simulink model "nonlinear_double_track_model"  (R2024b)
settings: Solver=VariableStepAuto  StartTime=0.0  StopTime=5  FixedStep=0.01  RelTol=1e-4
undefined symbols (not defined in the file or in its folder): a, b, balance_bar_ratio_front, brake_pedal_motion_ratio, cg_height, disc_radius_front, disc_radius_rear, front_mass_distribution, g, gear_ratio, Jeq, Ki_yaw, Kp_yaw, mass_total, max_pedal_force, mc_diameter_front, mc_diameter_rear, num_pistons_front, num_pistons_rear, pad_friction_front, pad_friction_rear, pad_height_front, pad_height_rear, piston_radius_front, piston_radius_rear, static_toe_front, static_toe_rear, tire_loaded_radius, tire_mu, tire_mu_correction_factor, tlltd, toe_deflection_rear, track_width_front, track_width_rear, wheelbase, yaw_polar_inertia
libraries: simulink_extras x12, checkpacklib x1, slpidlib x1
(part 1 of 6)
settings Model: Creator=bglen  LastModifiedBy=37rst
settings RTWCC: GPUComputeCapability=3.5

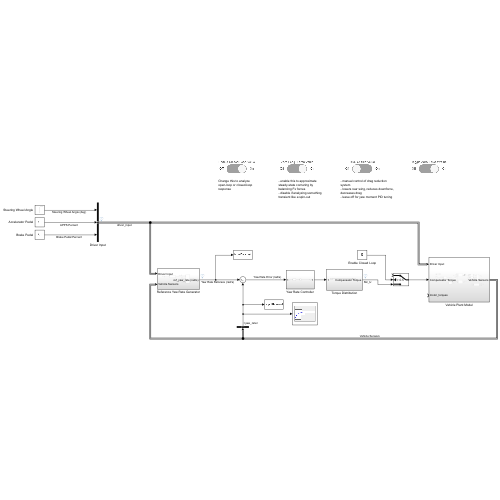
[thumbnail: Simulink's stored preview of the top-level diagram]
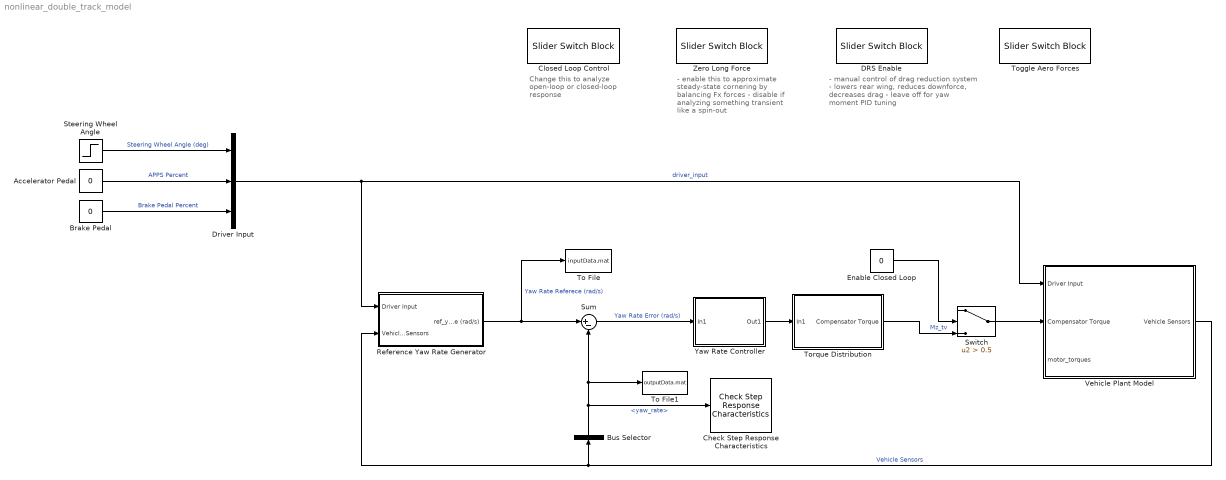
== system nonlinear_double_track_model ==
  note: - enable this to approximate
      | steady-state cornering by
      | balancing Fx forces
      | - disable if analyzing something
      | transient like a spin-out
  note: Change this to analyze
      | open-loop or closed-loop
      | response
  note: - manual control of drag reduction
      | system
      | - lowers rear wing, reduces downforce,
      | decreases drag
      | - leave off for yaw moment PID tuning
  Accelerator Pedal : Constant  Value=0
  Brake Pedal : Constant  Value=0
  Bus Selector : BusSelector  OutputSignals=yaw_rate
  Check Step Response Characteristics : checkpacklib/Check Step Response  Characteristics  InstantiateOnLoad=on  enabled=on  stopWhenAssertionFail=off  export=off  ViewDlgPos=[]  OpenViewOnLoad=off  LaunchViewOnOpen=off  TimeUnits=seconds  EnableStepResponseBound=on  InitialValue=0  FinalValue=.426  StepTime=1  RiseTime=1  PercentRise=80  SettlingTime=2  PercentSettling=1  PercentOvershoot=10  PercentUndershoot=1
      settings: LibrarySourceBlock=|
      | sdolib/Signal Constraints/Check Step Response
      | Characteristics
  Closed Loop Control : SliderSwitchBlock
      settings: Values={{'Off', 'On'}, [1 0]}
  DRS Enable : SliderSwitchBlock
      settings: Values={{'Off', 'On'}, [0 1]}
  Driver Input : BusCreator  Inputs=3
  Enable Closed Loop : Constant  Value=0
  Reference Yaw Rate Generator : Subsystem
  Steering Wheel Angle : Step  After=90  SampleTime=0
  Sum : Sum  Inputs=|+-
  Switch : Switch  Criteria=u2 > Threshold  Threshold=0.5  SaturateOnIntegerOverflow=off  InputSameDT=off
  To File : ToFile  Filename=inputData.mat  MatrixName=inputData
  To File1 : ToFile  Filename=outputData.mat  MatrixName=outputData
  Toggle Aero Forces : SliderSwitchBlock
      settings: Values={{'Off', 'On'}, [0 -1]}
  Torque Distribution : Subsystem
  Vehicle Plant Model : Subsystem
  Yaw Rate Controller : Subsystem
  Zero Long Force : SliderSwitchBlock
      settings: Values={{'Off', 'On'}, [0 1]}
  wires:
  Sum/1 -> Yaw Rate Controller/1(In1)  "Yaw Rate Error (rad/s)"
  Accelerator Pedal/1 -> Driver Input/2  "APPS Percent"
  Steering Wheel Angle/1 -> Driver Input/1  "Steering Wheel Angle (deg)"
  Brake Pedal/1 -> Driver Input/3  "Brake Pedal Percent"
  Driver Input/1 -> Reference Yaw Rate Generator/1(Driver Input), Vehicle Plant Model/1(Driver Input)  "driver_input"
  Vehicle Plant Model/1(Vehicle Sensors) -> Reference Yaw Rate Generator/2(Vehicle Sensors), Bus Selector/1  "Vehicle Sensors"
  Bus Selector/1 -> Check Step Response Characteristics/1, Sum/2, To File1/1  "<yaw_rate>"
  Yaw Rate Controller/1(Out1) -> Torque Distribution/1(In1)
  Reference Yaw Rate Generator/1(ref_yaw_rate (rad/s)) -> To File/1, Sum/1  "Yaw Rate Referece (rad/s)"
  Switch/1 -> Vehicle Plant Model/2(Compensator Torque)
  Torque Distribution/1(Compensator Torque) -> Switch/3  "Mz_tv"
  Enable Closed Loop/1 -> Switch/2

== system nonlinear_double_track_model/Reference Yaw Rate Generator ==
  Driver Input : Inport
  Vehicle Sensors : Inport  Port=2
  Bus Selector : BusSelector  OutputSignals=body_velocity_x
  Bus Selector1 : BusSelector  OutputSignals=Steering Wheel Angle (deg)
  MATLAB Function : MATLAB Function  TreatAsAtomicUnit=on
  ZR25 Steering System : Subsystem
  g : Constant  Value=g
  l : Constant  Value=wheelbase
  mu : Constant  Value=tire_mu
  sigma : Constant  Value=tire_mu_correction_factor
  ref_yaw_rate (rad/s) : Outport
  wires:
  MATLAB Function/1(ref_yaw_rate) -> ref_yaw_rate (rad/s)/1
  mu/1 -> MATLAB Function/3(mu)
  sigma/1 -> MATLAB Function/4(sigma)
  l/1 -> MATLAB Function/5(wheelbase)
  g/1 -> MATLAB Function/6(g)
  Vehicle Sensors/1 -> Bus Selector/1
  Bus Selector/1 -> MATLAB Function/1(velocity)  "<body_velocity_x>"
  Driver Input/1 -> Bus Selector1/1
  Bus Selector1/1 -> ZR25 Steering System/1(Steering input at the rack)  "<Steering Wheel Angle (deg)>"
  ZR25 Steering System/1(Steering Angles (rad)) -> MATLAB Function/2(steer_angles)  "Front Steering Angles (rad)"

== system nonlinear_double_track_model/Torque Distribution ==
  In1 : Inport
  Compensator Torque : Outport
  wires:
  In1/1 -> Compensator Torque/1

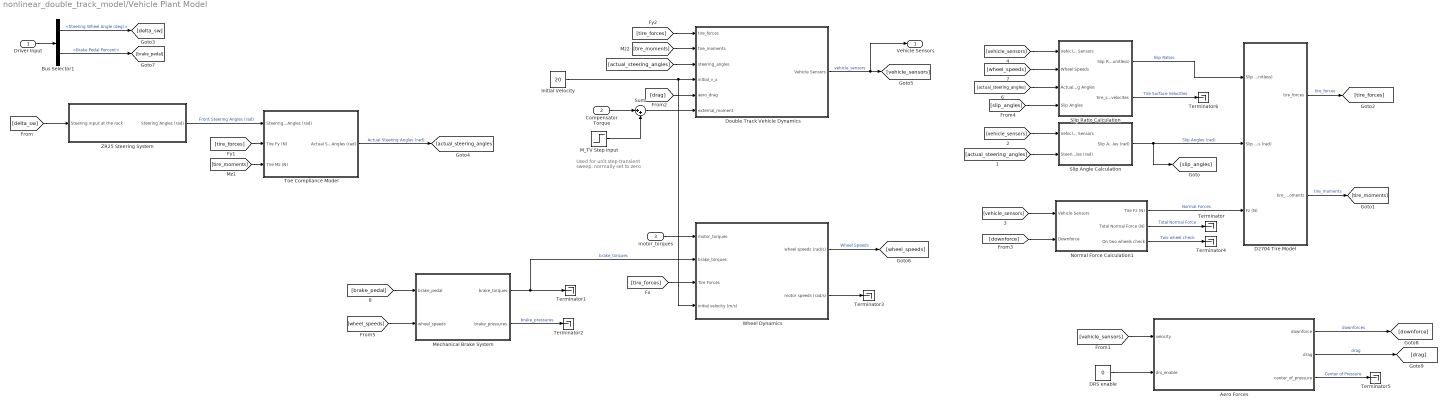
== system nonlinear_double_track_model/Vehicle Plant Model ==
  note: Used for unit step transient sweep, normally set to zero
  Driver Input : Inport
  Compensator Torque : Inport  Port=2
  motor_torques : Inport  Port=3
  Aero Forces : Subsystem
      mask Display: image('big_wing.png');
  Bus Selector1 : BusSelector  OutputSignals=Steering Wheel Angle (deg),Brake Pedal Percent
  D2704 Tire Model : Subsystem
  DRS enable : Constant  Value=0
  Double Track Vehicle Dynamics : Subsystem
      mask Display: image('double_track_diagram.png');
  Initial Velocity : Constant  Value=20
  M_TV Step Input : Step  After=0  SampleTime=0
  Mechanical Brake System : Subsystem
  Normal Force Calculation1 : Subsystem
  Slip Angle Calculation : Subsystem
  Slip Ratio Calculation : Subsystem
  Sum : Sum  Inputs=|++
  Terminator{,1..6} (7 alike) : Terminator
  Toe Compliance Model : Subsystem
  Wheel Dynamics : Subsystem
  ZR25 Steering System : Subsystem
  Vehicle Sensors : Outport
  wires:
  Slip Angle Calculation/1(Slip Angles (rad)) -> [slip_angles], D2704 Tire Model/2(Slip Angles (rad))  "Slip Angles (rad)"
  Normal Force Calculation1/2(Total Normal Force (N)) -> Terminator/1  "Total Normal Force"
  Normal Force Calculation1/1(Tire Fz (N)) -> D2704 Tire Model/3(Fz (N))  "Normal Forces"
  ZR25 Steering System/1(Steering Angles (rad)) -> Toe Compliance Model/1(Steering Angles (rad))  "Front Steering Angles (rad)"
  Initial Velocity/1 -> Wheel Dynamics/4(initial velocity (m/s)), Double Track Vehicle Dynamics/4(initial_v_x)
  Normal Force Calculation1/3(On two wheels check) -> Terminator4/1  "Two wheel check"
  D2704 Tire Model/2(tire_moments) -> [tire_moments]  "tire_moments"
  [tire_moments] -> Double Track Vehicle Dynamics/2(tire_moments)
  D2704 Tire Model/1(tire_forces) -> [tire_forces]  "tire_forces"
  [tire_forces] -> Double Track Vehicle Dynamics/1(tire_forces)
  Driver Input/1 -> Bus Selector1/1
  Bus Selector1/1 -> [delta_sw]  "<Steering Wheel Angle (deg)>"
  [delta_sw] -> ZR25 Steering System/1(Steering input at the rack)
  M_TV Step Input/1 -> Sum/2
  Compensator Torque/1 -> Sum/1
  Sum/1 -> Double Track Vehicle Dynamics/6(external_moment)
  Toe Compliance Model/1(Actual Steering Angles (rad)) -> [actual_steering_angles]  "Actual Steering Angles (rad)"
  [tire_forces] -> Toe Compliance Model/2(Tire Fy (N))
  [tire_moments] -> Toe Compliance Model/3(Tire Mz (N))
  [actual_steering_angles] -> Double Track Vehicle Dynamics/3(steering_angles)
  [actual_steering_angles] -> Slip Angle Calculation/2(Steering Angles (rad))
  [vehicle_sensors] -> Slip Angle Calculation/1(Vehicle Sensors)
  [vehicle_sensors] -> Normal Force Calculation1/1(Vehicle Sensors)
  [vehicle_sensors] -> Slip Ratio Calculation/1(Vehicle Sensors)
  Double Track Vehicle Dynamics/1(Vehicle Sensors) -> Vehicle Sensors/1, [vehicle_sensors]  "vehicle_sensors"
  [actual_steering_angles] -> Slip Ratio Calculation/3(Actual Steering Angles)
  Wheel Dynamics/1(wheel speeds (rad/s)) -> [wheel_speeds]  "Wheel Speeds"
  [wheel_speeds] -> Slip Ratio Calculation/2(Wheel Speeds)
  motor_torques/1 -> Wheel Dynamics/1(motor_torques)
  Bus Selector1/2 -> [brake_pedal]  "<Brake Pedal Percent>"
  [brake_pedal] -> Mechanical Brake System/1(brake_pedal)
  Mechanical Brake System/1(brake_torques) -> Wheel Dynamics/2(brake_torques), Terminator1/1  "brake_torques"
  Mechanical Brake System/2(brake_pressures) -> Terminator2/1  "brake_pressures"
  Wheel Dynamics/2(motor speeds (rad/s)) -> Terminator3/1
  Aero Forces/1(downforce) -> [downforce]  "downforces"
  [vehicle_sensors] -> Aero Forces/1(velocity)
  DRS enable/1 -> Aero Forces/2(drs_enable)
  Aero Forces/2(drag) -> [drag]  "drag"
  Aero Forces/3(center_of_pressure) -> Terminator5/1  "Center of Pressure"
  [drag] -> Double Track Vehicle Dynamics/5(aero_drag)
  [downforce] -> Normal Force Calculation1/2(Downforce)
  [slip_angles] -> Slip Ratio Calculation/4(Slip Angles)
  Slip Ratio Calculation/2(tire_surface_velocites) -> Terminator6/1  "Tire Surface Velocities"
  Slip Ratio Calculation/1(Slip Ratios (unitless)) -> D2704 Tire Model/1(Slip Ratios (unitless))  "Slip Ratios"
  [tire_forces] -> Wheel Dynamics/3(Tire Forces)
  [wheel_speeds] -> Mechanical Brake System/2(wheel_speeds)

== system nonlinear_double_track_model/Yaw Rate Controller ==
  In1 : Inport
  PID Controller : slpidlib/PID Controller  Controller=PI  Form=Parallel  TimeDomain=Continuous-time  UseExternalTs=off  SampleTime=-1  IntegratorMethod=Forward Euler  FilterMethod=Forward Euler  ControllerParametersSource=internal  P=Kp_yaw  I=Ki_yaw  UseKiTs=off  D=0  UseExternalDerivativeSource=off  N=100  UseFilter=on  TunerSelectOption=Transfer Function Based (PID Tuner App)  InitialConditionSource=internal  InitialConditionForIntegrator=0  InitialConditionForFilter=0  DifferentiatorICPrevScaledInput=0  ExternalReset=none  TrackingMode=off  Kt=1  LimitOutput=off  SatLimitsSource=internal  UpperSaturationLimit=7000  LowerSaturationLimit=-7000  LinearizeAsGain=on  AntiWindupMode=back-calculation  Kb=1  LimitIntegrator=off  UpperIntegratorSaturationLimit=inf  LowerIntegratorSaturationLimit=-inf  POutMin=[]  POutMax=[]  IOutMin=[]  IOutMax=[]  DOutMin=[]  DOutMax=[]  NOutMin=[]  NOutMax=[]  SumOutMin=[]  SumOutMax=[]  SaturationOutMin=[]  SaturationOutMax=[]  PParamMin=[]  PParamMax=[]  IParamMin=[]  IParamMax=[]  DParamMin=[]  DParamMax=[]  NParamMin=[]  NParamMax=[]  KbParamMin=[]  KbParamMax=[]  KtParamMin=[]  KtParamMax=[]  KbOutMin=[]  KbOutMax=[]  KtOutMin=[]  KtOutMax=[]  IntegratorOutMin=[]  IntegratorOutMax=[]  FilterOutMin=[]  FilterOutMax=[]  SumI1OutMin=[]  SumI1OutMax=[]  SumI2OutMin=[]  SumI2OutMax=[]  SumI3OutMin=[]  SumI3OutMax=[]  SumI4OutMin=[]  SumI4OutMax=[]  SumDOutMin=[]  SumDOutMax=[]  DifferentiatorOutMin=[]  DifferentiatorOutMax=[]  FilterDiffOutCoefMin=[]  FilterDiffOutCoefMax=[]  ReciprocalOutMin=[]  ReciprocalOutMax=[]  SumDenOutMin=[]  SumDenOutMax=[]  SumNumOutMin=[]  SumNumOutMax=[]  DivideOutMin=[]  DivideOutMax=[]  UdiffTsProdOutMin=[]  UdiffTsProdOutMax=[]  NTsProdOutMin=[]  NTsProdOutMax=[]  UintegralTsProdOutMin=[]  UintegralTsProdOutMax=[]  UngainTsProdOutMin=[]  UngainTsProdOutMax=[]  ClampingZeroOutDataTypeStr=Inherit: Inherit via back propagation  ClampingZeroOutMin=[]  ClampingZeroOutMax=[]  FilterDenConstantOutDataTypeStr=Inherit: Inherit via back propagation  FilterDenConstantOutMin=[]  FilterDenConstantOutMax=[]  PCopyOutDataTypeStr=Inherit: Inherit via back propagation  PCopyOutMin=[]  PCopyOutMax=[]  NCopyOutDataTypeStr=Inherit: Inherit via back propagation  NCopyOutMin=[]  NCopyOutMax=[]  IntegratorContinuousStateAttributes=''  IntegratorStateMustResolveToSignalObject=off  IntegratorRTWStateStorageClass=Auto  FilterContinuousStateAttributes=''  FilterStateMustResolveToSignalObject=off  FilterRTWStateStorageClass=Auto  ParallelPVariant=InternalParameters  IdealPVariant=Disabled  IVariant=InternalParameters  DVariant=Disabled  IntegratorVariant=Continuous  SatVariant=Disabled  AWVariant=Passthrough  PCopyVariant=Disabled  TRVariant=Disabled  FdbkBlocksVariant=Forward  IdealPFdbkVariant=Disabled  SatFdbkVariant=Disabled  DerivativeFilterVariant=Disabled  NVariant=Disabled  NCopyVariant=Disabled_SignalSpec  FilterICVariant=Disabled  IntegratorICVariant=InternalICs  ExternalResetVariant=Disabled  TRSumVariant=Passthrough  SumFdbkVariant=Disabled  SumVariant=Sum_PI  TsampFilterVariant=InternalTs  TsampNgainVariant=Passthrough  TsampIntegralVariant=Disabled_SignalSpec  ExternalSaturationOutDataTypeStr=Inherit: Same as second input  DerivativeSourceVariant=Disabled  PreIntVariant=InternalPreInt
  Saturation : Saturate  UpperLimit=7000  LowerLimit=-7000
  Out1 : Outport
  wires:
  In1/1 -> PID Controller/1
  PID Controller/1 -> Saturation/1
  Saturation/1 -> Out1/1

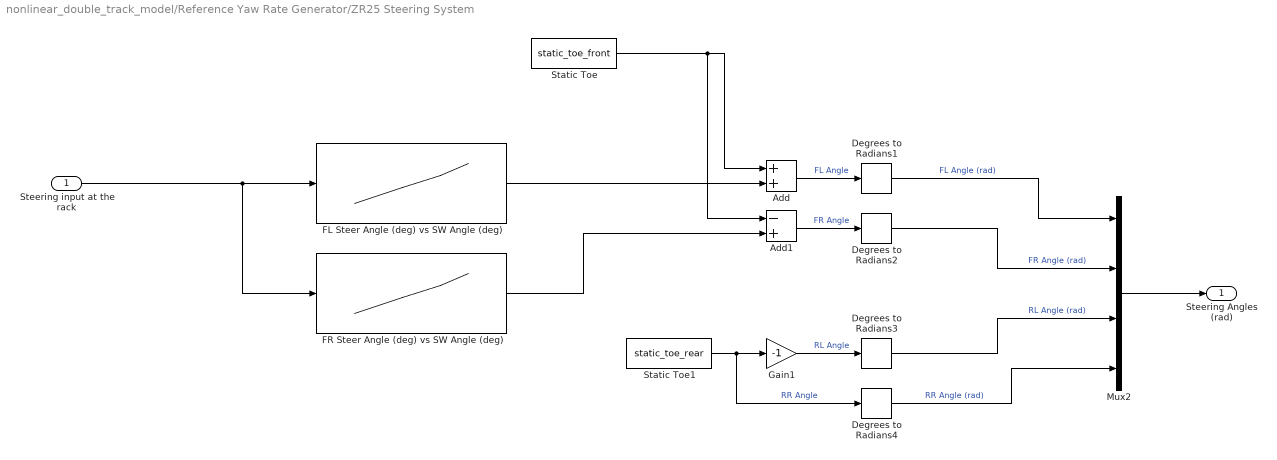
== system nonlinear_double_track_model/Reference Yaw Rate Generator/ZR25 Steering System ==
  Steering input at the rack : Inport
  Add : Sum
  Add1 : Sum  Inputs=-+
  Degrees to Radians{1..4} (4 alike) : simulink_extras/Transformations/Degrees to Radians
  FL Steer Angle (deg) vs SW Angle (deg) : Lookup_n-D  NumberOfTableDimensions=1  BreakpointsForDimension1=[-180;-162;-144;-126;-108;-90;-72;-54;-36;-18;0;18;36;54;72;90;108;126;144;162;180]  Table=[-37.683;-33.549;-29.57;-25.708;-21.934;-18.224;-14.558;-10.918;-7.289;-3.654;0;3.689;7.428;11.234;15.126;19.127;23.261;27.563;32.073;36.847;41.964]  InternalRulePriority=Speed  RndMeth=Simplest
  FR Steer Angle (deg) vs SW Angle (deg) : Lookup_n-D  NumberOfTableDimensions=1  BreakpointsForDimension1=[-180;-162;-144;-126;-108;-90;-72;-54;-36;-18;0;18;36;54;72;90;108;126;144;162;180]  Table=[-41.964;-36.847;-32.073;-27.563;-23.261;-19.126;-15.126;-11.234;-7.428;-3.689;0;3.654;7.289;10.918;14.558;18.225;21.934;25.708;29.57;33.549;37.683]  InternalRulePriority=Speed  RndMeth=Simplest
  Gain1 : Gain  Gain=-1
  Mux2 : Mux
  Static Toe : Constant  Value=static_toe_front
  Static Toe1 : Constant  Value=static_toe_rear
  Steering Angles (rad) : Outport
  wires:
  Steering input at the rack/1 -> FR Steer Angle (deg) vs SW Angle (deg)/1, FL Steer Angle (deg) vs SW Angle (deg)/1
  Add1/1 -> Degrees to Radians2/1  "FR Angle"
  Add/1 -> Degrees to Radians1/1  "FL Angle"
  Static Toe/1 -> Add/1, Add1/1
  Static Toe1/1 -> Gain1/1, Degrees to Radians4/1  "RR Angle"
  Gain1/1 -> Degrees to Radians3/1  "RL Angle"
  Degrees to Radians1/1 -> Mux2/1  "FL Angle (rad)"
  Degrees to Radians2/1 -> Mux2/2  "FR Angle (rad)"
  Degrees to Radians3/1 -> Mux2/3  "RL Angle (rad)"
  Degrees to Radians4/1 -> Mux2/4  "RR Angle (rad)"
  Mux2/1 -> Steering Angles (rad)/1
  FL Steer Angle (deg) vs SW Angle (deg)/1 -> Add/2
  FR Steer Angle (deg) vs SW Angle (deg)/1 -> Add1/2

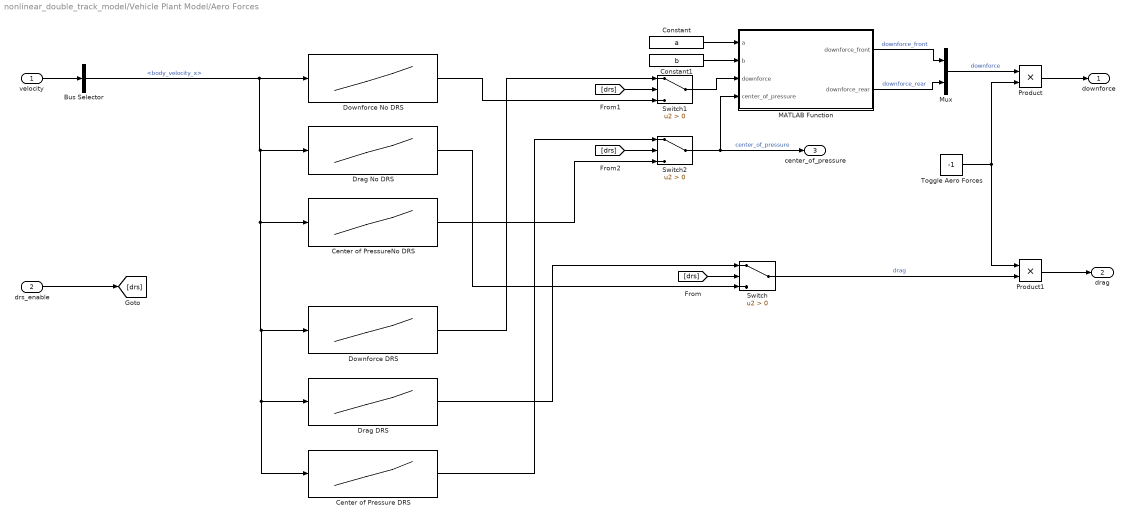
== system nonlinear_double_track_model/Vehicle Plant Model/Aero Forces ==
  velocity : Inport
  drs_enable : Inport  Port=2
  Bus Selector : BusSelector  OutputSignals=body_velocity_x
  Center of Pressure DRS : Lookup_n-D  NumberOfTableDimensions=1  BreakpointsForDimension1=[2.2352;4.4704;6.7056;8.9408;11.176;13.4112;15.6464;17.8816;20.1168;22.352;24.5872;26.8224;29.0576;31.2928]  Table=[0.176794972966434;0.172602682700208;0.0934978495226155;0.109635046481717;0.0961822849769587;0.0578976665904581;-0.0518352816153028;-0.0850922821311282;-0.112456802684495;-0.110386434440698;-0.121870663573613;-0.172151238551353;-0.185862061581233;-0.199113354149404]  InternalRulePriority=Speed  RndMeth=Simplest
  Center of PressureNo DRS : Lookup_n-D  NumberOfTableDimensions=1  BreakpointsForDimension1=[2.2352;4.4704;6.7056;8.9408;11.176;13.4112;15.6464;17.8816;20.1168;22.352;24.5872;26.8224;29.0576;31.2928]  Table=[-0.135607188802615;-0.137588787703048;-0.195574365979447;-0.215866639322259;-0.22870367751938;-0.278157751621036;-0.366989667896679;-0.310089315236121;-0.333799351635467;-0.289895228213292;-0.377134768583161;-0.440956951229536;-0.50107873320069;-0.516936678234731]  InternalRulePriority=Speed  RndMeth=Simplest
  Constant : Constant  Value=a
  Constant1 : Constant  Value=b
  Downforce DRS : Lookup_n-D  NumberOfTableDimensions=1  BreakpointsForDimension1=[2.2352;4.4704;6.7056;8.9408;11.176;13.4112;15.6464;17.8816;20.1168;22.352;24.5872;26.8224;29.0576;31.2928]  Table=[48.732728255261;71.2374454240421;99.6302490967395;113.50202079035;144.789613578015;217.750213991217;279.051769333153;479.898861584762;694.616494558787;799.328698956136;849.239673042502;860.401352372326;898.870223824537;946.94400741494]  InternalRulePriority=Speed  RndMeth=Simplest
  Downforce No DRS : Lookup_n-D  NumberOfTableDimensions=1  BreakpointsForDimension1=[2.2352;4.4704;6.7056;8.9408;11.176;13.4112;15.6464;17.8816;20.1168;22.352;24.5872;26.8224;29.0576;31.2928]  Table=[67.415259039521;98.5940083581371;133.792591102243;157.005628187984;200.837205930795;313.238609177833;401.822685915433;596.334521302368;902.755723164394;939.369924924251;1159.7623600173;1240.39910819797;1454.89792573704;1551.90214709574]  InternalRulePriority=Speed  RndMeth=Simplest
  Drag DRS : Lookup_n-D  NumberOfTableDimensions=1  BreakpointsForDimension1=[2.2352;4.4704;6.7056;8.9408;11.176;13.4112;15.6464;17.8816;20.1168;22.352;24.5872;26.8224;29.0576;31.2928]  Table=[19.6993853490744;27.57897401486;63.0372609057655;90.6162349206255;118.195208935485;181.232469841251;275.7897401486;334.8087445404;374.274256355665;401.853230370525;571.27086774381;642.195715217825;886.470961759645;984.95547796671]  InternalRulePriority=Speed  RndMeth=Simplest
  Drag No DRS : Lookup_n-D  NumberOfTableDimensions=1  BreakpointsForDimension1=[2.2352;4.4704;6.7056;8.9408;11.176;13.4112;15.6464;17.8816;20.1168;22.352;24.5872;26.8224;29.0576;31.2928]  Table=[22.2411080765;31.1375513071;71.1715458448;102.3090971519;133.446648459;209.0664159191;311.375513071;355.857729224;422.5810534535;453.7186047606;533.786593836;578.268809989;711.715458448;1112.055403825]  InternalRulePriority=Speed  RndMeth=Simplest
  MATLAB Function : MATLAB Function  TreatAsAtomicUnit=on
  Mux : Mux  Inputs=2
  Product : Product
  Product1 : Product
  Switch : Switch  Criteria=u2 > Threshold  SaturateOnIntegerOverflow=off  InputSameDT=off
  Switch1 : Switch  Criteria=u2 > Threshold  SaturateOnIntegerOverflow=off  InputSameDT=off
  Switch2 : Switch  Criteria=u2 > Threshold  SaturateOnIntegerOverflow=off  InputSameDT=off
  Toggle Aero Forces : Constant  Value=-1
  downforce : Outport
  drag : Outport  Port=2
  center_of_pressure : Outport  Port=3
  wires:
  Constant1/1 -> MATLAB Function/2(b)
  Constant/1 -> MATLAB Function/1(a)
  MATLAB Function/1(downforce_front) -> Mux/1  "downforce_front"
  MATLAB Function/2(downforce_rear) -> Mux/2  "downforce_rear"
  Mux/1 -> Product/1  "downforce"
  Switch/1 -> Product1/2  "drag"
  Drag No DRS/1 -> Switch/3
  Drag DRS/1 -> Switch/1
  [drs] -> Switch/2
  [drs] -> Switch1/2
  [drs] -> Switch2/2
  Switch1/1 -> MATLAB Function/3(downforce)
  Downforce No DRS/1 -> Switch1/3
  Downforce DRS/1 -> Switch1/1
  Center of PressureNo DRS/1 -> Switch2/3
  Center of Pressure DRS/1 -> Switch2/1
  drs_enable/1 -> [drs]
  Switch2/1 -> MATLAB Function/4(center_of_pressure), center_of_pressure/1  "center_of_pressure"
  velocity/1 -> Bus Selector/1
  Bus Selector/1 -> Center of Pressure DRS/1, Drag DRS/1, Downforce DRS/1, Center of PressureNo DRS/1, Drag No DRS/1, Downforce No DRS/1  "<body_velocity_x>"
  Product1/1 -> drag/1
  Product/1 -> downforce/1
  Toggle Aero Forces/1 -> Product1/1, Product/2

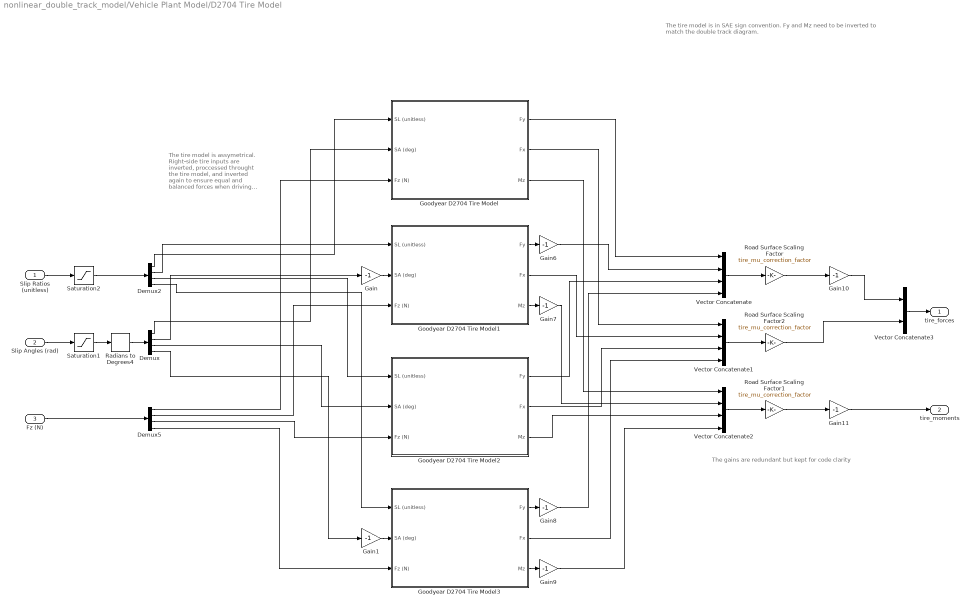
== system nonlinear_double_track_model/Vehicle Plant Model/D2704 Tire Model ==
  note: The gains are redundant but kept for code clarity
  note: The tire model is assymetrical. Right-side tire inputs are inverted, proccessed throught the tire model, and inverted again to ensure equal and balanced forces when driving straight.
  note: The tire model is in SAE sign convention. Fy and Mz need to be inverted to match the double track diagram.
  Slip Ratios (unitless) : Inport
  Slip Angles (rad) : Inport  Port=2
  Fz (N) : Inport  Port=3
  Demux : Demux
  Demux2 : Demux
  Demux5 : Demux
  Gain{,1,6..11} (8 alike) : Gain  Gain=-1
  Goodyear D2704 Tire Model : Subsystem
  Goodyear D2704 Tire Model1 : Subsystem
  Goodyear D2704 Tire Model2 : Subsystem
  Goodyear D2704 Tire Model3 : Subsystem
  Radians to Degrees4 : simulink_extras/Transformations/Radians to Degrees
  Road Surface Scaling Factor : Gain  Gain=tire_mu_correction_factor
  Road Surface Scaling Factor1 : Gain  Gain=tire_mu_correction_factor
  Road Surface Scaling Factor2 : Gain  Gain=tire_mu_correction_factor
  Saturation1 : Saturate  UpperLimit=0.261  LowerLimit=-0.261
  Saturation2 : Saturate  UpperLimit=0.3  LowerLimit=-0.3
  Vector Concatenate : Concatenate  NumInputs=4  Mode=Multidimensional array  ConcatenateDimension=2
  Vector Concatenate1 : Concatenate  NumInputs=4  Mode=Multidimensional array  ConcatenateDimension=2
  Vector Concatenate2 : Concatenate  NumInputs=4  Mode=Multidimensional array  ConcatenateDimension=2
  Vector Concatenate3 : Concatenate  Mode=Multidimensional array
  tire_forces : Outport
  tire_moments : Outport  Port=2
  wires:
  Slip Angles (rad)/1 -> Saturation1/1
  Vector Concatenate/1 -> Road Surface Scaling Factor/1
  Demux/2 -> Gain/1
  Demux/4 -> Gain1/1
  Vector Concatenate2/1 -> Road Surface Scaling Factor1/1
  Road Surface Scaling Factor1/1 -> Gain11/1
  Saturation1/1 -> Radians to Degrees4/1
  Slip Ratios (unitless)/1 -> Saturation2/1
  Saturation2/1 -> Demux2/1
  Vector Concatenate1/1 -> Road Surface Scaling Factor2/1
  Road Surface Scaling Factor2/1 -> Vector Concatenate3/2
  Goodyear D2704 Tire Model3/2(Fx) -> Vector Concatenate1/4
  Goodyear D2704 Tire Model1/2(Fx) -> Vector Concatenate1/2
  Radians to Degrees4/1 -> Demux/1
  Goodyear D2704 Tire Model3/1(Fy) -> Gain8/1
  Goodyear D2704 Tire Model1/1(Fy) -> Gain6/1
  Goodyear D2704 Tire Model2/1(Fy) -> Vector Concatenate/3
  Goodyear D2704 Tire Model/1(Fy) -> Vector Concatenate/1
  Goodyear D2704 Tire Model1/3(Mz) -> Gain7/1
  Goodyear D2704 Tire Model3/3(Mz) -> Gain9/1
  Goodyear D2704 Tire Model2/2(Fx) -> Vector Concatenate1/3
  Goodyear D2704 Tire Model/2(Fx) -> Vector Concatenate1/1
  Goodyear D2704 Tire Model2/3(Mz) -> Vector Concatenate2/3
  Goodyear D2704 Tire Model/3(Mz) -> Vector Concatenate2/1
  Fz (N)/1 -> Demux5/1
  Demux2/1 -> Goodyear D2704 Tire Model/1(SL (unitless))
  Demux2/2 -> Goodyear D2704 Tire Model1/1(SL (unitless))
  Demux2/3 -> Goodyear D2704 Tire Model2/1(SL (unitless))
  Demux2/4 -> Goodyear D2704 Tire Model3/1(SL (unitless))
  Demux/1 -> Goodyear D2704 Tire Model/2(SA (deg))
  Gain/1 -> Goodyear D2704 Tire Model1/2(SA (deg))
  Demux/3 -> Goodyear D2704 Tire Model2/2(SA (deg))
  Gain1/1 -> Goodyear D2704 Tire Model3/2(SA (deg))
  Demux5/1 -> Goodyear D2704 Tire Model/3(Fz (N))
  Demux5/2 -> Goodyear D2704 Tire Model1/3(Fz (N))
  Demux5/3 -> Goodyear D2704 Tire Model2/3(Fz (N))
  Demux5/4 -> Goodyear D2704 Tire Model3/3(Fz (N))
  Vector Concatenate3/1 -> tire_forces/1
  Road Surface Scaling Factor/1 -> Gain10/1
  Gain6/1 -> Vector Concatenate/2
  Gain8/1 -> Vector Concatenate/4
  Gain7/1 -> Vector Concatenate2/2
  Gain9/1 -> Vector Concatenate2/4
  Gain10/1 -> Vector Concatenate3/1
  Gain11/1 -> tire_moments/1
